simulink model "wlan_phy_rx_pmd"  (Simulink 7.8)
settings: Solver=VariableStepDiscrete  SolverMode=SingleTasking  StartTime=0.0  StopTime=rx_sim.sim_time  RelTol=1e-3
PostLoadFcn:
  | wlan_phy_rx_init
InitFcn:
  | wlan_phy_rx_init
StartFcn:
  | wlan_phy_rx_init
values from neighbouring files (symbols this model uses):
  barker_seq20 = 0.95 * circshift(barker_seq20, [0 4]) ./ max(abs(barker_seq20))    [wlan_phy_rx_init.m]
  bit_scrambler_lfsr_bytes = sum(bit_scrambler_lfsr_bytes .* repmat(2.^[0:7], 127, 1),2)    [wlan_phy_rx_init.m]
  longCorr_coef_i = [3*real(longCorr_coef)]    [wlan_phy_rx_init.m]
  longCorr_coef_q = [3*imag(longCorr_coef)]    [wlan_phy_rx_init.m]
  MAX_CP_LEN = 32    [wlan_phy_rx_init.m]
  MAX_NCBPS = NCBPS_11n_64QAM    (= 312)    [wlan_phy_rx_init.m]
  MAX_NUM_BYTES = 2^16-1    (= 65535)    [wlan_phy_rx_init.m]
  MAX_NUM_SC = 64    [wlan_phy_rx_init.m]
  MAX_NUM_SYMS = 600    [wlan_phy_rx_init.m]
  NDBPS_11a_BPSK12 = 24    [wlan_phy_rx_init.m]
  preFFT_sampBuff_numSamps = 4*MAX_NUM_SC    (= 256)    [wlan_phy_rx_init.m]
  rx_sim = struct()    [wlan_phy_rx_init.m]
  scr_ind_rev = zeros(1,128)    [wlan_phy_rx_init.m]
undefined symbols (not defined in the file or in its folder): gcbh, mcs_rom_11ag, mcs_rom_11n, wlan_rx_deinterleave_rom_gen, xlBlockLoadCallback, xlGetNormalizedPeriod, xlipgetpartsetting
libraries: xbsIndex_r4 x4943, xbsEDKLib_r4 x1
(part 4 of 28)

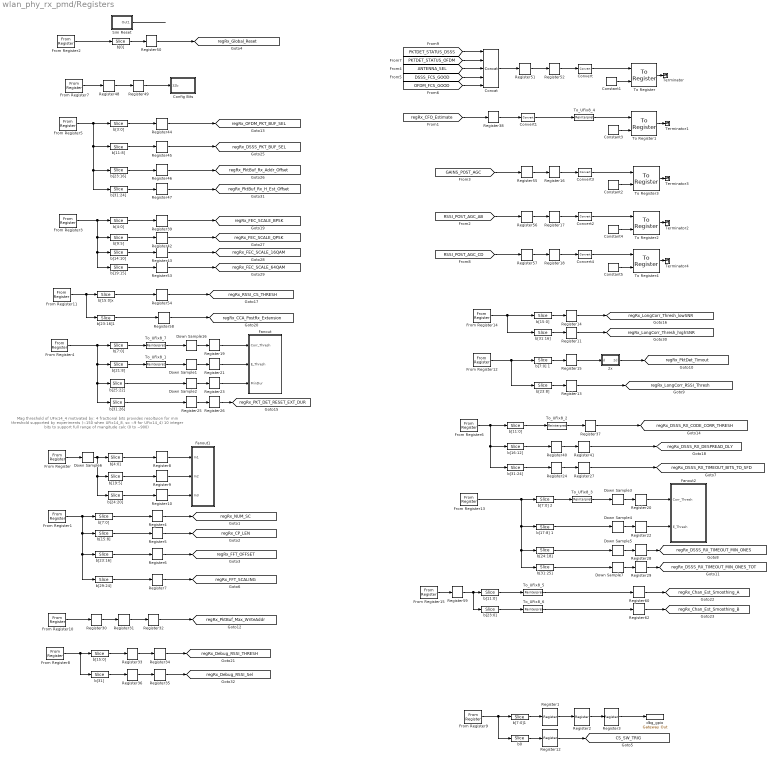
== system wlan_phy_rx_pmd/Registers ==
  note: Mag threshold of UFix14_4 motivated by:
      | 4 fractional bits provides resoltuion for min threshold supported by experiments (~150 when UFix14_8, so ~9 for UFix14_4)
      | 10 integer bits to support full range of mangitude calc (0 to ~900)
  " b[23:16] " : xbsIndex_r4/Slice  nbits=8  boolean_output=off  mode=Lower Bit Location + Width  bit1=0  base1=MSB of Input  bit0=16  base0=LSB of Input  dbl_ovrd=off  block_type=slice
      settings: block_version=VER_STRING_GOES_HERE
  " b[31:24]" : xbsIndex_r4/Slice  nbits=8  boolean_output=off  mode=Lower Bit Location + Width  bit1=0  base1=MSB of Input  bit0=24  base0=LSB of Input  dbl_ovrd=off  block_type=slice
      settings: block_version=VER_STRING_GOES_HERE
  "2x " : Subsystem
  Concat : xbsIndex_r4/Concat  num_inputs=5  dbl_ovrd=off  block_type=concat
      settings: block_version=8.2.02
  Config Bits : Subsystem
  Constant{1..5} (5 alike) : xbsIndex_r4/Constant  const=1  gui_display_data_type=Boolean  arith_type=Boolean  n_bits=30  bin_pt=0  preci_type=Single  exp_width=8  frac_width=24  explicit_period=on  period=1  equ=P=C  opselect=C  inp2=PCIN>>17  opr=+  inp1=P  carry=CIN  dbl_ovrd=off  block_type=constant
  Convert{,2..4} (4 alike) : xbsIndex_r4/Convert  gui_display_data_type=Fixed-point  arith_type=Unsigned  n_bits=32  bin_pt=0  float_type=Single  exp_bits=8  fraction_bits=24  quantization=Truncate  overflow=Wrap  en=off  latency=0  dbl_ovrd=off  pipeline=off  block_type=convert
  Convert1 : xbsIndex_r4/Convert  gui_display_data_type=Fixed-point  arith_type="Signed  (2's comp)"  n_bits=32  bin_pt=31  float_type=Single  exp_bits=8  fraction_bits=24  quantization=Truncate  overflow=Wrap  en=off  latency=0  dbl_ovrd=off  pipeline=off  block_type=convert
  Down Sample{1..5,7,16} (7 alike) : xbsIndex_r4/Down Sample  sample_ratio=8  sample_phase="Last Value of Frame  (most efficient)"  en=off  latency=1  dbl_ovrd=off  block_type=dsamp
      settings: block_version=VER_STRING_GOES_HERE
  Down Sample6 : xbsIndex_r4/Down Sample  sample_ratio=16  sample_phase="Last Value of Frame  (most efficient)"  en=off  latency=1  dbl_ovrd=off  block_type=dsamp
      settings: block_version=VER_STRING_GOES_HERE
  Fanout : Subsystem
  Fanout1 : Subsystem
  Fanout2 : Subsystem
  From Register : xbsIndex_r4/From Register  shared_memory_name='PKTDET_RSSI_CONFIG'  init=REG_RX_PKTDET_RSSI_CONFIG  period=1  ownership=Locally owned and initialized  gui_display_data_type=Fixed-point  arith_type=Unsigned  n_bits=32  bin_pt=0  preci_type=Single  dbl_ovrd=off  block_type=fromreg
      settings: AttributesFormatString=<< %<shared_memory_name> >>  infoedit="Register block that reads data to a shared memory register.  Delay of one sample period."
  From Register1 : xbsIndex_r4/From Register  shared_memory_name='FFT_Config'  init=REG_RX_FFT_Config  period=1  ownership=Locally owned and initialized  gui_display_data_type=Fixed-point  arith_type=Unsigned  n_bits=32  bin_pt=0  preci_type=Single  dbl_ovrd=off  block_type=fromreg
      settings: AttributesFormatString=<< %<shared_memory_name> >>  infoedit="Register block that reads data to a shared memory register.  Delay of one sample period."
  From Register10 : xbsIndex_r4/From Register  shared_memory_name='PKTBUF_MAX_WRITE_ADDR'  init=REG_RX_PKT_BUF_Max_Write_Addr  period=1  ownership=Locally owned and initialized  gui_display_data_type=Fixed-point  arith_type=Unsigned  n_bits=32  bin_pt=0  preci_type=Single  dbl_ovrd=off  block_type=fromreg
      settings: AttributesFormatString=<< %<shared_memory_name> >>  infoedit="Register block that reads data to a shared memory register.  Delay of one sample period."
  From Register11 : xbsIndex_r4/From Register  shared_memory_name='PHY_CCA_CONFIG'  init=REG_RX_CCA_CONFIG  period=1  ownership=Locally owned and initialized  gui_display_data_type=Fixed-point  arith_type=Unsigned  n_bits=32  bin_pt=0  preci_type=Single  dbl_ovrd=off  block_type=fromreg
      settings: AttributesFormatString=<< %<shared_memory_name> >>  infoedit="Register block that reads data to a shared memory register.  Delay of one sample period."
  From Register12 : xbsIndex_r4/From Register  shared_memory_name='LTS_Corr_Config'  init=REG_RX_LTS_Corr_Confg  period=1  ownership=Locally owned and initialized  gui_display_data_type=Fixed-point  arith_type=Unsigned  n_bits=32  bin_pt=0  preci_type=Single  dbl_ovrd=off  block_type=fromreg
      settings: AttributesFormatString=<< %<shared_memory_name> >>  infoedit="Register block that reads data to a shared memory register.  Delay of one sample period."
  From Register13 : xbsIndex_r4/From Register  shared_memory_name='PKTDET_DSSS_CONFIG'  init=REG_RX_PktDet_DSSS_Config  period=1  ownership=Locally owned and initialized  gui_display_data_type=Fixed-point  arith_type=Unsigned  n_bits=32  bin_pt=0  preci_type=Single  dbl_ovrd=off  block_type=fromreg
      settings: AttributesFormatString=<< %<shared_memory_name> >>  infoedit="Register block that reads data to a shared memory register.  Delay of one sample period."
  From Register14 : xbsIndex_r4/From Register  shared_memory_name='LTS_Corr_Thresh'  init=REG_RX_LTS_Corr_Thresh  period=1  ownership=Locally owned and initialized  gui_display_data_type=Fixed-point  arith_type=Unsigned  n_bits=32  bin_pt=0  preci_type=Single  dbl_ovrd=off  block_type=fromreg
      settings: AttributesFormatString=<< %<shared_memory_name> >>  infoedit="Register block that reads data to a shared memory register.  Delay of one sample period."
  From Register15 : xbsIndex_r4/From Register  shared_memory_name='CHAN_EST_SMOOTHING'  init=REG_RX_Chan_Est_Smoothing  period=1  ownership=Locally owned and initialized  gui_display_data_type=Fixed-point  arith_type=Unsigned  n_bits=32  bin_pt=0  preci_type=Single  dbl_ovrd=off  block_type=fromreg
      settings: AttributesFormatString=<< %<shared_memory_name> >>  infoedit="Register block that reads data to a shared memory register.  Delay of one sample period."
  From Register2 : xbsIndex_r4/From Register  shared_memory_name='Control'  init=REG_RX_Control  period=1  ownership=Locally owned and initialized  gui_display_data_type=Fixed-point  arith_type=Unsigned  n_bits=32  bin_pt=0  preci_type=Single  dbl_ovrd=off  block_type=fromreg
      settings: AttributesFormatString=<< %<shared_memory_name> >>  infoedit="Register block that reads data to a shared memory register.  Delay of one sample period."
  From Register3 : xbsIndex_r4/From Register  shared_memory_name='FEC_CONFIG'  init=REG_RX_FEC_Config  period=1  ownership=Locally owned and initialized  gui_display_data_type=Fixed-point  arith_type=Unsigned  n_bits=32  bin_pt=0  preci_type=Single  dbl_ovrd=off  block_type=fromreg
      settings: AttributesFormatString=<< %<shared_memory_name> >>  infoedit="Register block that reads data to a shared memory register.  Delay of one sample period."
  From Register4 : xbsIndex_r4/From Register  shared_memory_name='PKTDET_AUTOCORR_CONFIG'  init=REG_RX_PktDet_AutoCorr_Config  period=1  ownership=Locally owned and initialized  gui_display_data_type=Fixed-point  arith_type=Unsigned  n_bits=32  bin_pt=0  preci_type=Single  dbl_ovrd=off  block_type=fromreg
      settings: AttributesFormatString=<< %<shared_memory_name> >>  infoedit="Register block that reads data to a shared memory register.  Delay of one sample period."
  From Register5 : xbsIndex_r4/From Register  shared_memory_name='PKT_BUF_SEL'  init=REG_RX_PktBuf_Sel  period=1  ownership=Locally owned and initialized  gui_display_data_type=Fixed-point  arith_type=Unsigned  n_bits=32  bin_pt=0  preci_type=Single  dbl_ovrd=off  block_type=fromreg
      settings: AttributesFormatString=<< %<shared_memory_name> >>  infoedit="Register block that reads data to a shared memory register.  Delay of one sample period."
  From Register6 : xbsIndex_r4/From Register  shared_memory_name='DSSS_RX_CONFIG'  init=REG_RX_DSSS_RX_CONFIG  period=1  ownership=Locally owned and initialized  gui_display_data_type=Fixed-point  arith_type=Unsigned  n_bits=32  bin_pt=0  preci_type=Single  dbl_ovrd=off  block_type=fromreg
      settings: AttributesFormatString=<< %<shared_memory_name> >>  infoedit="Register block that reads data to a shared memory register.  Delay of one sample period."
  From Register7 : xbsIndex_r4/From Register  shared_memory_name='CONFIG'  init=REG_RX_Config  period=1  ownership=Locally owned and initialized  gui_display_data_type=Fixed-point  arith_type=Unsigned  n_bits=32  bin_pt=0  preci_type=Single  dbl_ovrd=off  block_type=fromreg
      settings: AttributesFormatString=<< %<shared_memory_name> >>  infoedit="Register block that reads data to a shared memory register.  Delay of one sample period."
  From Register8 : xbsIndex_r4/From Register  shared_memory_name='RSSI_THRESH'  init=600  period=1  ownership=Locally owned and initialized  gui_display_data_type=Fixed-point  arith_type=Unsigned  n_bits=32  bin_pt=0  preci_type=Single  dbl_ovrd=off  block_type=fromreg
      settings: AttributesFormatString=<< %<shared_memory_name> >>  infoedit="Register block that reads data to a shared memory register.  Delay of one sample period."
  From Register9 : xbsIndex_r4/From Register  shared_memory_name='DEBUG_GPIO'  init=0  period=1  ownership=Locally owned and initialized  gui_display_data_type=Fixed-point  arith_type=Unsigned  n_bits=32  bin_pt=0  preci_type=Single  dbl_ovrd=off  block_type=fromreg
      settings: AttributesFormatString=<< %<shared_memory_name> >>  infoedit="Register block that reads data to a shared memory register.  Delay of one sample period."
  Register{1..60,62} (61 alike) : xbsIndex_r4/Register  init=0  rst=off  en=off  dbl_ovrd=off  block_type=register
  Sim Reset : Subsystem
  Terminator{,1..4} (5 alike) : Terminator
  To Register : xbsIndex_r4/To Register  shared_memory_name='Status'  init=0  ownership=Locally owned and initialized  explicit_data_type=on  gui_display_data_type=Fixed-point  arith_type=Unsigned  n_bits=32  bin_pt=0  preci_type=Single  dbl_ovrd=off  block_type=toreg
      settings: AttributesFormatString=<< %<shared_memory_name> >>  infoedit="Register block that writes data to a shared memory register.  Delay of one sample period."
  To Register1 : xbsIndex_r4/To Register  shared_memory_name='CFO_EST_TIME_DOMAIN'  init=0  ownership=Locally owned and initialized  explicit_data_type=on  gui_display_data_type=Fixed-point  arith_type=Unsigned  n_bits=32  bin_pt=0  preci_type=Single  dbl_ovrd=off  block_type=toreg
      settings: AttributesFormatString=<< %<shared_memory_name> >>  infoedit="Register block that writes data to a shared memory register.  Delay of one sample period."
  To Register2 : xbsIndex_r4/To Register  shared_memory_name='RX_PKT_RSSI_AB'  init=0  ownership=Locally owned and initialized  explicit_data_type=on  gui_display_data_type=Fixed-point  arith_type=Unsigned  n_bits=32  bin_pt=0  preci_type=Single  dbl_ovrd=off  block_type=toreg
      settings: AttributesFormatString=<< %<shared_memory_name> >>  infoedit="Register block that writes data to a shared memory register.  Delay of one sample period."
  To Register3 : xbsIndex_r4/To Register  shared_memory_name='RX_PKT_AGC_GAINS'  init=0  ownership=Locally owned and initialized  explicit_data_type=on  gui_display_data_type=Fixed-point  arith_type=Unsigned  n_bits=32  bin_pt=0  preci_type=Single  dbl_ovrd=off  block_type=toreg
      settings: AttributesFormatString=<< %<shared_memory_name> >>  infoedit="Register block that writes data to a shared memory register.  Delay of one sample period."
  To Register4 : xbsIndex_r4/To Register  shared_memory_name='RX_PKT_RSSI_CD'  init=0  ownership=Locally owned and initialized  explicit_data_type=on  gui_display_data_type=Fixed-point  arith_type=Unsigned  n_bits=32  bin_pt=0  preci_type=Single  dbl_ovrd=off  block_type=toreg
      settings: AttributesFormatString=<< %<shared_memory_name> >>  infoedit="Register block that writes data to a shared memory register.  Delay of one sample period."
  To_UFix8_1 : xbsIndex_r4/Reinterpret  force_arith_type=on  arith_type=Unsigned  force_bin_pt=on  bin_pt=4  block_type=cast
      settings: block_version=VER_STRING_GOES_HERE
  To_UFix8_2 : xbsIndex_r4/Reinterpret  force_arith_type=on  arith_type=Unsigned  force_bin_pt=on  bin_pt=4  block_type=cast
      settings: block_version=VER_STRING_GOES_HERE
  To_UFix8_3 : xbsIndex_r4/Reinterpret  force_arith_type=on  arith_type=Unsigned  force_bin_pt=on  bin_pt=6  block_type=cast
      settings: block_version=VER_STRING_GOES_HERE
  To_UFix8_4 : xbsIndex_r4/Reinterpret  force_arith_type=on  arith_type=Unsigned  force_bin_pt=on  bin_pt=0  block_type=cast
      settings: block_version=VER_STRING_GOES_HERE
  To_UFix8_5 : xbsIndex_r4/Reinterpret  force_arith_type=on  arith_type=Unsigned  force_bin_pt=on  bin_pt=12  block_type=cast
      settings: block_version=VER_STRING_GOES_HERE
  To_UFix8_6 : xbsIndex_r4/Reinterpret  force_arith_type=on  arith_type=Unsigned  force_bin_pt=on  bin_pt=12  block_type=cast
      settings: block_version=VER_STRING_GOES_HERE
  To_UFix8_7 : xbsIndex_r4/Reinterpret  force_arith_type=on  arith_type=Unsigned  force_bin_pt=on  bin_pt=8  block_type=cast
      settings: block_version=VER_STRING_GOES_HERE
  b0 : xbsIndex_r4/Slice  nbits=1  boolean_output=off  mode=Lower Bit Location + Width  bit1=0  base1=MSB of Input  bit0=0  base0=LSB of Input  dbl_ovrd=off  block_type=slice
      settings: block_version=VER_STRING_GOES_HERE
  b[0] : xbsIndex_r4/Slice  nbits=1  boolean_output=off  mode=Lower Bit Location + Width  bit1=0  base1=MSB of Input  bit0=0  base0=LSB of Input  dbl_ovrd=off  block_type=slice
      settings: block_version=VER_STRING_GOES_HERE
  b[11:0] : xbsIndex_r4/Slice  nbits=12  boolean_output=off  mode=Lower Bit Location + Width  bit1=0  base1=MSB of Input  bit0=0  base0=LSB of Input  dbl_ovrd=off  block_type=slice
      settings: block_version=VER_STRING_GOES_HERE
  "b[11:0]   " : xbsIndex_r4/Slice  nbits=12  boolean_output=off  mode=Lower Bit Location + Width  bit1=0  base1=MSB of Input  bit0=0  base0=LSB of Input  dbl_ovrd=off  block_type=slice
      settings: block_version=VER_STRING_GOES_HERE
  b[11:8] : xbsIndex_r4/Slice  nbits=4  boolean_output=off  mode=Lower Bit Location + Width  bit1=0  base1=MSB of Input  bit0=8  base0=LSB of Input  dbl_ovrd=off  block_type=slice
      settings: block_version=VER_STRING_GOES_HERE
  b[14:10] : xbsIndex_r4/Slice  nbits=5  boolean_output=off  mode=Lower Bit Location + Width  bit1=0  base1=MSB of Input  bit0=10  base0=LSB of Input  dbl_ovrd=off  block_type=slice
      settings: block_version=VER_STRING_GOES_HERE
  b[15:0] : xbsIndex_r4/Slice  nbits=16  boolean_output=off  mode=Lower Bit Location + Width  bit1=0  base1=MSB of Input  bit0=0  base0=LSB of Input  dbl_ovrd=off  block_type=slice
      settings: block_version=VER_STRING_GOES_HERE
  "b[15:0]    " : xbsIndex_r4/Slice  nbits=16  boolean_output=off  mode=Lower Bit Location + Width  bit1=0  base1=MSB of Input  bit0=0  base0=LSB of Input  dbl_ovrd=off  block_type=slice
      settings: block_version=VER_STRING_GOES_HERE
  b[15:0]x : xbsIndex_r4/Slice  nbits=16  boolean_output=off  mode=Lower Bit Location + Width  bit1=0  base1=MSB of Input  bit0=0  base0=LSB of Input  dbl_ovrd=off  block_type=slice
      settings: block_version=VER_STRING_GOES_HERE
  b[15:8] : xbsIndex_r4/Slice  nbits=8  boolean_output=off  mode=Lower Bit Location + Width  bit1=0  base1=MSB of Input  bit0=8  base0=LSB of Input  dbl_ovrd=off  block_type=slice
      settings: block_version=VER_STRING_GOES_HERE
  "b[16:12]   " : xbsIndex_r4/Slice  nbits=5  boolean_output=off  mode=Lower Bit Location + Width  bit1=0  base1=MSB of Input  bit0=12  base0=LSB of Input  dbl_ovrd=off  block_type=slice
      settings: block_version=VER_STRING_GOES_HERE
  b[17:8] 1 : xbsIndex_r4/Slice  nbits=10  boolean_output=off  mode=Lower Bit Location + Width  bit1=0  base1=MSB of Input  bit0=8  base0=LSB of Input  dbl_ovrd=off  block_type=slice
      settings: block_version=VER_STRING_GOES_HERE
  b[19:15] : xbsIndex_r4/Slice  nbits=5  boolean_output=off  mode=Lower Bit Location + Width  bit1=0  base1=MSB of Input  bit0=15  base0=LSB of Input  dbl_ovrd=off  block_type=slice
      settings: block_version=VER_STRING_GOES_HERE
  b[19:5] : xbsIndex_r4/Slice  nbits=15  boolean_output=off  mode=Lower Bit Location + Width  bit1=0  base1=MSB of Input  bit0=5  base0=LSB of Input  dbl_ovrd=off  block_type=slice
      settings: block_version=VER_STRING_GOES_HERE
  "b[21:8] " : xbsIndex_r4/Slice  nbits=14  boolean_output=off  mode=Lower Bit Location + Width  bit1=0  base1=MSB of Input  bit0=8  base0=LSB of Input  dbl_ovrd=off  block_type=slice
      settings: block_version=VER_STRING_GOES_HERE
  b[23:0] : xbsIndex_r4/Slice  nbits=12  boolean_output=off  mode=Lower Bit Location + Width  bit1=0  base1=MSB of Input  bit0=12  base0=LSB of Input  dbl_ovrd=off  block_type=slice
      settings: block_version=VER_STRING_GOES_HERE
  b[23:16] : xbsIndex_r4/Slice  nbits=8  boolean_output=off  mode=Lower Bit Location + Width  bit1=0  base1=MSB of Input  bit0=16  base0=LSB of Input  dbl_ovrd=off  block_type=slice
      settings: block_version=VER_STRING_GOES_HERE
  b[23:16]1 : xbsIndex_r4/Slice  nbits=8  boolean_output=off  mode=Lower Bit Location + Width  bit1=0  base1=MSB of Input  bit0=16  base0=LSB of Input  dbl_ovrd=off  block_type=slice
      settings: block_version=VER_STRING_GOES_HERE
  b[23:8] : xbsIndex_r4/Slice  nbits=16  boolean_output=off  mode=Lower Bit Location + Width  bit1=0  base1=MSB of Input  bit0=8  base0=LSB of Input  dbl_ovrd=off  block_type=slice
      settings: block_version=VER_STRING_GOES_HERE
  b[24:18] : xbsIndex_r4/Slice  nbits=7  boolean_output=off  mode=Lower Bit Location + Width  bit1=0  base1=MSB of Input  bit0=18  base0=LSB of Input  dbl_ovrd=off  block_type=slice
      settings: block_version=VER_STRING_GOES_HERE
  b[24:20] : xbsIndex_r4/Slice  nbits=5  boolean_output=off  mode=Lower Bit Location + Width  bit1=0  base1=MSB of Input  bit0=20  base0=LSB of Input  dbl_ovrd=off  block_type=slice
      settings: block_version=VER_STRING_GOES_HERE
  "b[25:22] " : xbsIndex_r4/Slice  nbits=4  boolean_output=off  mode=Lower Bit Location + Width  bit1=0  base1=MSB of Input  bit0=22  base0=LSB of Input  dbl_ovrd=off  block_type=slice
      settings: block_version=VER_STRING_GOES_HERE
  b[29:24] : xbsIndex_r4/Slice  nbits=6  boolean_output=off  mode=Lower Bit Location + Width  bit1=0  base1=MSB of Input  bit0=24  base0=LSB of Input  dbl_ovrd=off  block_type=slice
      settings: block_version=VER_STRING_GOES_HERE
  b[31:16] : xbsIndex_r4/Slice  nbits=16  boolean_output=off  mode=Lower Bit Location + Width  bit1=0  base1=MSB of Input  bit0=16  base0=LSB of Input  dbl_ovrd=off  block_type=slice
      settings: block_version=VER_STRING_GOES_HERE
  b[31:24] : xbsIndex_r4/Slice  nbits=8  boolean_output=off  mode=Lower Bit Location + Width  bit1=0  base1=MSB of Input  bit0=24  base0=LSB of Input  dbl_ovrd=off  block_type=slice
      settings: block_version=VER_STRING_GOES_HERE
  b[31:25] : xbsIndex_r4/Slice  nbits=7  boolean_output=off  mode=Lower Bit Location + Width  bit1=0  base1=MSB of Input  bit0=25  base0=LSB of Input  dbl_ovrd=off  block_type=slice
      settings: block_version=VER_STRING_GOES_HERE
  b[31:26] : xbsIndex_r4/Slice  nbits=6  boolean_output=off  mode=Lower Bit Location + Width  bit1=0  base1=MSB of Input  bit0=26  base0=LSB of Input  dbl_ovrd=off  block_type=slice
      settings: block_version=VER_STRING_GOES_HERE
  b[31] : xbsIndex_r4/Slice  nbits=1  boolean_output=off  mode=Lower Bit Location + Width  bit1=0  base1=MSB of Input  bit0=31  base0=LSB of Input  dbl_ovrd=off  block_type=slice
      settings: block_version=VER_STRING_GOES_HERE
  b[3:0] : xbsIndex_r4/Slice  nbits=4  boolean_output=off  mode=Lower Bit Location + Width  bit1=0  base1=MSB of Input  bit0=0  base0=LSB of Input  dbl_ovrd=off  block_type=slice
      settings: block_version=VER_STRING_GOES_HERE
  b[4:0] : xbsIndex_r4/Slice  nbits=5  boolean_output=off  mode=Lower Bit Location + Width  bit1=0  base1=MSB of Input  bit0=0  base0=LSB of Input  dbl_ovrd=off  block_type=slice
      settings: block_version=VER_STRING_GOES_HERE
  "b[4:0]  " : xbsIndex_r4/Slice  nbits=5  boolean_output=off  mode=Lower Bit Location + Width  bit1=0  base1=MSB of Input  bit0=0  base0=LSB of Input  dbl_ovrd=off  block_type=slice
      settings: block_version=VER_STRING_GOES_HERE
  b[7:0] : xbsIndex_r4/Slice  nbits=8  boolean_output=off  mode=Lower Bit Location + Width  bit1=0  base1=MSB of Input  bit0=0  base0=LSB of Input  dbl_ovrd=off  block_type=slice
      settings: block_version=VER_STRING_GOES_HERE
  "b[7:0] " : xbsIndex_r4/Slice  nbits=8  boolean_output=off  mode=Lower Bit Location + Width  bit1=0  base1=MSB of Input  bit0=0  base0=LSB of Input  dbl_ovrd=off  block_type=slice
      settings: block_version=VER_STRING_GOES_HERE
  b[7:0] 1 : xbsIndex_r4/Slice  nbits=8  boolean_output=off  mode=Lower Bit Location + Width  bit1=0  base1=MSB of Input  bit0=0  base0=LSB of Input  dbl_ovrd=off  block_type=slice
      settings: block_version=VER_STRING_GOES_HERE
  b[7:0] 2 : xbsIndex_r4/Slice  nbits=8  boolean_output=off  mode=Lower Bit Location + Width  bit1=0  base1=MSB of Input  bit0=0  base0=LSB of Input  dbl_ovrd=off  block_type=slice
      settings: block_version=VER_STRING_GOES_HERE
  b[7:0]1 : xbsIndex_r4/Slice  nbits=8  boolean_output=off  mode=Lower Bit Location + Width  bit1=0  base1=MSB of Input  bit0=0  base0=LSB of Input  dbl_ovrd=off  block_type=slice
      settings: block_version=VER_STRING_GOES_HERE
  b[9:5] : xbsIndex_r4/Slice  nbits=5  boolean_output=off  mode=Lower Bit Location + Width  bit1=0  base1=MSB of Input  bit0=5  base0=LSB of Input  dbl_ovrd=off  block_type=slice
      settings: block_version=VER_STRING_GOES_HERE
  dbg_gpio : xbsIndex_r4/Gateway Out  inherit_from_input=off  hdl_port=on  timing_constraint=None  locs_specified=off  LOCs={}  UseAsDAC=off  DACChannel='1'  block_type=gatewayout
  tag scope: CS_SW_TRIG=global, regRx_CCA_PostRx_Extension=global, regRx_CP_LEN=global, regRx_Chan_Est_Smoothing_A=global, regRx_Chan_Est_Smoothing_B=global, regRx_DSSS_PKT_BUF_SEL=global, regRx_DSSS_RX_CODE_CORR_THRESH=global, regRx_DSSS_RX_DESPREAD_DLY=global, regRx_DSSS_RX_TIMEOUT_BITS_TO_SFD=global, regRx_DSSS_RX_TIMEOUT_MIN_ONES=global, regRx_DSSS_RX_TIMEOUT_MIN_ONES_TOT=global, regRx_Debug_RSSI_Sel=global, regRx_Debug_RSSI_THRESH=global, regRx_FEC_SCALE_16QAM=global, regRx_FEC_SCALE_64QAM=global, regRx_FEC_SCALE_BPSK=global, regRx_FEC_SCALE_QPSK=global, regRx_FFT_OFFSET=global, regRx_FFT_SCALING=global, regRx_Global_Reset=global, regRx_LongCorr_RSSI_Thresh=global, regRx_LongCorr_Thresh_highSNR=global, regRx_LongCorr_Thresh_lowSNR=global, regRx_NUM_SC=global, regRx_OFDM_PKT_BUF_SEL=global, regRx_PKT_DET_RESET_EXT_DUR=global, regRx_PktBuf_Max_WriteAddr=global, regRx_PktBuf_Rx_Addr_Offset=global, regRx_PktBuf_Rx_H_Est_Offset=global, regRx_PktDet_Timout=global, regRx_RSSI_CS_THRESH=global
  wires:
  b[31:16]/1 -> Register11/1
  From Register1/1 -> b[7:0]/1, b[15:8]/1, b[23:16]/1, b[29:24]/1
  b[7:0]/1 -> Register4/1
  b[15:8]/1 -> Register5/1
  b[23:16]/1 -> Register6/1
  From Register2/1 -> b[0]/1
  b[0]/1 -> Register50/1
  From Register3/1 -> "b[4:0]  "/1, b[9:5]/1, b[14:10]/1, b[19:15]/1
  b[29:24]/1 -> Register7/1
  [DSSS_FCS_GOOD] -> Concat/4
  [OFDM_FCS_GOOD] -> Concat/5
  Constant1/1 -> To Register/2
  To Register/1 -> Terminator/1
  From Register4/1 -> "b[7:0] "/1, "b[21:8] "/1, "b[25:22] "/1, b[31:26]/1
  "b[7:0] "/1 -> To_UFix8_7/1
  "b[21:8] "/1 -> To_UFix8_1/1
  To_UFix8_7/1 -> Down Sample16/1
  To_UFix8_1/1 -> Down Sample1/1
  Down Sample16/1 -> Register19/1
  Down Sample1/1 -> Register21/1
  b[15:0]/1 -> Register14/1
  "b[25:22] "/1 -> Down Sample2/1
  Down Sample2/1 -> Register23/1
  Sim Reset/1(Out1) -> ?
  From Register5/1 -> b[3:0]/1, b[11:8]/1, " b[23:16] "/1, " b[31:24]"/1
  b[3:0]/1 -> Register44/1
  From Register6/1 -> "b[11:0]   "/1, "b[16:12]   "/1, b[31:24]/1
  "b[11:0]   "/1 -> To_UFix8_2/1
  To_UFix8_2/1 -> Register37/1
  From Register8/1 -> "b[15:0]    "/1, b[31]/1
  "b[15:0]    "/1 -> Register33/1
  From Register9/1 -> b[7:0]1/1, b0/1
  b[7:0]1/1 -> Register1/1
  Register1/1 -> Register2/1
  Register2/1 -> Register3/1
  Register3/1 -> dbg_gpio/1
  b[4:0]/1 -> Register8/1
  Down Sample6/1 -> b[4:0]/1, b[19:5]/1, b[24:20]/1
  b[19:5]/1 -> Register9/1
  From Register/1 -> Down Sample6/1
  b[24:20]/1 -> Register10/1
  From Register7/1 -> Register48/1
  Register4/1 -> [regRx_NUM_SC]
  Register5/1 -> [regRx_CP_LEN]
  Register6/1 -> [regRx_FFT_OFFSET]
  Register7/1 -> [regRx_FFT_SCALING]
  Register8/1 -> Fanout1/1(In1)
  Register9/1 -> Fanout1/2(In2)
  Register10/1 -> Fanout1/3(In3)
  Register11/1 -> [regRx_LongCorr_Thresh_highSNR]
  b[31:26]/1 -> Register25/1
  From Register11/1 -> b[15:0]x/1, b[23:16]1/1
  b[15:0]x/1 -> Register54/1
  b[23:16]1/1 -> Register58/1
  b[11:8]/1 -> Register45/1
  Convert/1 -> To Register/1
  " b[23:16] "/1 -> Register46/1
  "b[4:0]  "/1 -> Register39/1
  b[9:5]/1 -> Register42/1
  b[14:10]/1 -> Register43/1
  b0/1 -> Register12/1
  Register12/1 -> [CS_SW_TRIG]
  Constant4/1 -> To Register2/2
  To Register2/1 -> Terminator2/1
  Convert2/1 -> To Register2/1
  Register13/1 -> [regRx_LongCorr_RSSI_Thresh]
  b[23:8]/1 -> Register13/1
  Register14/1 -> [regRx_LongCorr_Thresh_lowSNR]
  From Register14/1 -> b[15:0]/1, b[31:16]/1
  From Register12/1 -> b[7:0] 1/1, b[23:8]/1
  "2x "/1(2d) -> [regRx_PktDet_Timout]
  Register15/1 -> "2x "/1(d)
  b[7:0] 1/1 -> Register15/1
  " b[31:24]"/1 -> Register47/1
  [RSSI_POST_AGC_AB] -> Register56/1
  [ANTENNA_SEL] -> Concat/3
  Constant2/1 -> To Register3/2
  To Register3/1 -> Terminator3/1
  Convert3/1 -> To Register3/1
  [GAINS_POST_AGC] -> Register55/1
  Register16/1 -> Convert3/1
  Register17/1 -> Convert2/1
  b[31]/1 -> Register36/1
  Concat/1 -> Register51/1
  [PKTDET_STATUS_OFDM] -> Concat/2
  Constant5/1 -> To Register4/2
  To Register4/1 -> Terminator4/1
  Convert4/1 -> To Register4/1
  Register18/1 -> Convert4/1
  [RSSI_POST_AGC_CD] -> Register57/1
  Register19/1 -> Fanout/1(Corr_Thresh)
  Register21/1 -> Fanout/2(E_Thresh)
  Register23/1 -> Fanout/3(MinDur)
  Register33/1 -> Register34/1
  Register34/1 -> [regRx_Debug_RSSI_THRESH]
  Register35/1 -> [regRx_Debug_RSSI_Sel]
  Register36/1 -> Register35/1
  Register37/1 -> [regRx_DSSS_RX_CODE_CORR_THRESH]
  Register40/1 -> Register41/1
  Register41/1 -> [regRx_DSSS_RX_DESPREAD_DLY]
  Register44/1 -> [regRx_OFDM_PKT_BUF_SEL]
  Register45/1 -> [regRx_DSSS_PKT_BUF_SEL]
  Register46/1 -> [regRx_PktBuf_Rx_Addr_Offset]
  Register47/1 -> [regRx_PktBuf_Rx_H_Est_Offset]
  Register48/1 -> Register49/1
  Register49/1 -> Config Bits/1(32b)
  Register50/1 -> [regRx_Global_Reset]
  Register51/1 -> Register52/1
  Register52/1 -> Convert/1
  Register55/1 -> Register16/1
  Register56/1 -> Register17/1
  Register57/1 -> Register18/1
  Register25/1 -> Register26/1
  Register26/1 -> [regRx_PKT_DET_RESET_EXT_DUR]
  From Register13/1 -> b[7:0] 2/1, b[17:8] 1/1, b[24:18]/1, b[31:25]/1
  b[7:0] 2/1 -> To_UFix8_3/1
  To_UFix8_3/1 -> Down Sample3/1
  Down Sample3/1 -> Register20/1
  Register20/1 -> Fanout2/1(Corr_Thresh)
  b[17:8] 1/1 -> Down Sample4/1
  Down Sample4/1 -> Register22/1
  Register22/1 -> Fanout2/2(E_Thresh)
  [PKTDET_STATUS_DSSS] -> Concat/1
  "b[16:12]   "/1 -> Register40/1
  b[31:24]/1 -> Register24/1
  Register24/1 -> Register27/1
  Register27/1 -> [regRx_DSSS_RX_TIMEOUT_BITS_TO_SFD]
  b[24:18]/1 -> Down Sample5/1
  Down Sample5/1 -> Register28/1
  Register28/1 -> [regRx_DSSS_RX_TIMEOUT_MIN_ONES]
  b[31:25]/1 -> Down Sample7/1
  Down Sample7/1 -> Register29/1
  Register29/1 -> [regRx_DSSS_RX_TIMEOUT_MIN_ONES_TOT]
  From Register10/1 -> Register30/1
  Register30/1 -> Register31/1
  Register31/1 -> Register32/1
  Register32/1 -> [regRx_PktBuf_Max_WriteAddr]
  [regRx_CFO_Estimate] -> Register38/1
  Constant3/1 -> To Register1/2
  To Register1/1 -> Terminator1/1
  To_UFix8_4/1 -> To Register1/1
  Convert1/1 -> To_UFix8_4/1
  Register38/1 -> Convert1/1
  b[19:15]/1 -> Register53/1
  Register39/1 -> [regRx_FEC_SCALE_BPSK]
  Register42/1 -> [regRx_FEC_SCALE_QPSK]
  Register43/1 -> [regRx_FEC_SCALE_16QAM]
  Register53/1 -> [regRx_FEC_SCALE_64QAM]
  Register54/1 -> [regRx_RSSI_CS_THRESH]
  Register58/1 -> [regRx_CCA_PostRx_Extension]
  From Register15/1 -> Register59/1
  Register59/1 -> b[11:0]/1, b[23:0]/1
  b[11:0]/1 -> To_UFix8_5/1
  b[23:0]/1 -> To_UFix8_6/1
  To_UFix8_5/1 -> Register60/1
  To_UFix8_6/1 -> Register62/1
  Register60/1 -> [regRx_Chan_Est_Smoothing_A]
  Register62/1 -> [regRx_Chan_Est_Smoothing_B]

== system wlan_phy_rx_pmd/Resets ==
  Convert1 : xbsIndex_r4/Convert  gui_display_data_type=Boolean  arith_type=Boolean  n_bits=16  bin_pt=14  float_type=Single  exp_bits=8  fraction_bits=24  quantization=Truncate  overflow=Wrap  en=off  latency=0  dbl_ovrd=off  pipeline=off  block_type=convert
  tag scope: RX_GLOBAL_RESET=global
  wires:
  Convert1/1 -> [RX_GLOBAL_RESET]
  [regRx_Global_Reset] -> Convert1/1

== system wlan_phy_rx_pmd/Sync & Antenna Sel ==
  Rx I : Inport
  Rx Q : Inport  Port=2
  IQ Valid : Inport  Port=3
  RSSI : Inport  Port=4
  "Reset " : Inport  Port=5
  ADC/Corr : Scope  NumInputPorts=10
      settings: TimeRange=13944  YMin=0.95~-1~-1~0.95~-1~-1~-1~-1~-1~-1  YMax=1.05~1~1~1.05~1~1~1~1~1~1  SaveName=ScopeData34
  Antenna Selection : Subsystem
  Debug  Outputs : Subsystem
  Gateway Out{,1..4} (5 alike) : xbsIndex_r4/Gateway Out  inherit_from_input=off  hdl_port=off  timing_constraint=None  locs_specified=off  LOCs={}  UseAsDAC=off  DACChannel='1'  block_type=gatewayout
  LTS Correlation : Subsystem
  PHY CCA : Subsystem
  Pkt Det : Subsystem
  Pkt Det AGC Ctrl : Subsystem
  Resets : Subsystem
  " Pkt Det DSSS" : Outport
  " Rx I" : Outport  Port=2
  " Rx Q" : Outport  Port=3
  " IQ Valid" : Outport  Port=4
  " Pkt Det OFDM" : Outport  Port=5
  LTS Sync : Outport  Port=6
  wires:
  LTS Correlation/1(Det) -> LTS Sync/1, PHY CCA/1(LTS Sync), Debug  Outputs/1(LTS)
  LTS Correlation/2(Timeout) -> PHY CCA/2(LTS Timeout), Resets/3(LTS Timeout), Debug  Outputs/2(LTS Timeout), Gateway Out2/1
  Gateway Out2/1 -> ADC/Corr/3  "LTS TO"
  Gateway Out1/1 -> ADC/Corr/2  "Pkt Done"
  Gateway Out/1 -> ADC/Corr/1  "Pkt Det OFDM"
  Pkt Det/1(Det OFDM) -> Antenna Selection/1(Pkt Det), LTS Correlation/5(En), PHY CCA/4(Det OFDM), Debug  Outputs/3(Det OFDM), Gateway Out/1, " Pkt Det OFDM"/1, Pkt Det AGC Ctrl/1(Det OFDM)
  Resets/1(" OFDM Reset") -> Antenna Selection/6(Reset), LTS Correlation/6(Reset), PHY CCA/6(Reset OFDM), Gateway Out1/1, Pkt Det/1(Reset OFDM)
  IQ Valid/1 -> Pkt Det/5(IQ Valid), Antenna Selection/4(IQ Valid)
  RSSI/1 -> Pkt Det/6(RSSI Vec), Antenna Selection/5(RSSI Vec), PHY CCA/5(Avg RSSI Vec)
  Rx I/1 -> Pkt Det/3(Rx I Vec), Antenna Selection/2(Rx I Vec)
  Rx Q/1 -> Pkt Det/4(Rx Q Vec), Antenna Selection/3(Rx Q Vec)
  Antenna Selection/1(Rx I) -> " Rx I"/1, LTS Correlation/1(rx_I)
  Antenna Selection/2(" Rx Q") -> " Rx Q"/1, LTS Correlation/2(rx_Q)
  Antenna Selection/3(" IQ Valid") -> " IQ Valid"/1, LTS Correlation/3(rx_IQ_valid), PHY CCA/3(IQ Valid)
  Antenna Selection/4(RSSI) -> LTS Correlation/4(RSSI), Gateway Out3/1  "Avg RSSI"
  Gateway Out3/1 -> ADC/Corr/9
  Pkt Det/2(Det DSSS) -> Gateway Out4/1, " Pkt Det DSSS"/1, Pkt Det AGC Ctrl/2(Det DSSS), Debug  Outputs/4(Det DSSS), PHY CCA/7(Det DSSS)
  Gateway Out4/1 -> ADC/Corr/4  "Pkt Det DSSS"
  Resets/2(DSSS Reset) -> Pkt Det/2(Reset DSSS), PHY CCA/8(Reset DSSS)
  [DSSS_RX_RESET] -> Resets/2("DSSS Reset ")
  "Reset "/1 -> Resets/1(OFDM Reset)

== system wlan_phy_rx_pmd/CFO & Samp Buffer/CFO Correction ==
  note: DDS requires >1 cycle EN early to get setup
      | Use gloabl reset for this
      | Safe, since DDS output is ignored when
      | it's not being used, so bogus outputs
      | between packets is no problem
      |
      | DDS updates once per input sample
      | Since the FFT only consumes 64 samples
      | per OFDM symbol (ignoring the CP),
      | the DDS keeps running after each FFT
      | frame is loaded. This is fine, since the FFT is
      | configured for non-streaming (it ignores its
      | I/Q inputs while computing a transform)
  "I " : Inport
  "Q " : Inport  Port=2
  DDS Run : Inport  Port=3
  Valid : Inport  Port=4
  tlast : Inport  Port=5
  CFO Est : Inport  Port=6
  CFO Corr : Scope  NumInputPorts=8
      settings: TimeRange=35000  YMin=0~-0.4~0~-1~0~0~-0.4~0  YMax=1~0.4~1~2~1~1~0.4~1  SaveName=ScopeData3
  "Complex Multiplier 5.0 " : xbsIndex_r4/Complex Multiplier 5.0   hasatlast=off  hasatuser=on  atuserwidth=1  hasbtlast=off  hasbtuser=off  btuserwidth=1  multtype=Use_Mults  optimizegoal=Performance  flowcontrol=NonBlocking  outputwidth=16  roundmode=Truncate  hasctrltlast=off  hasctrltuser=off  ctrltuserwidth=1  outtlastbehv=Null  latencyconfig=Manual  minimumlatency=2  aclken=off  aresetn=off  trim_axipin_name=on  aportwidth=63  bportwidth=63  ip_name=Complex Multiplier  ip_version=5.0  dsptool_ready=true  ipcore_usecache=true  ipcore_useipmodelcache=true  ipcore_verbose=false  ipcore_latency_parameter='minimumlatency'  wrapper_available=true  port_translation_map={ 'aclken' => 'en', 'aresetn' => 'rst'}  structural_sim=false  block_type=cmpy_v5_0
  Constant1 : xbsIndex_r4/Constant  const=1  gui_display_data_type=Boolean  arith_type=Boolean  n_bits=16  bin_pt=14  preci_type=Single  exp_width=8  frac_width=24  explicit_period=off  period=8  equ=P=C  opselect=C  inp2=PCIN>>17  opr=+  inp1=P  carry=CIN  dbl_ovrd=off  block_type=constant
  Constant2 : xbsIndex_r4/Constant  const=1  gui_display_data_type=Fixed-point  arith_type=Signed (2's comp)  n_bits=16  bin_pt=15  preci_type=Single  exp_width=8  frac_width=24  explicit_period=on  period=1  equ=P=C  opselect=C  inp2=PCIN>>17  opr=+  inp1=P  carry=CIN  dbl_ovrd=off  block_type=constant
  Constant3 : xbsIndex_r4/Constant  const=0  gui_display_data_type=Fixed-point  arith_type=Signed (2's comp)  n_bits=16  bin_pt=15  preci_type=Single  exp_width=8  frac_width=24  explicit_period=on  period=1  equ=P=C  opselect=C  inp2=PCIN>>17  opr=+  inp1=P  carry=CIN  dbl_ovrd=off  block_type=constant
  Constant5 : xbsIndex_r4/Constant  const=1  gui_display_data_type=Boolean  arith_type=Boolean  n_bits=16  bin_pt=14  preci_type=Single  exp_width=8  frac_width=24  explicit_period=off  period=8  equ=P=C  opselect=C  inp2=PCIN>>17  opr=+  inp1=P  carry=CIN  dbl_ovrd=off  block_type=constant
  Convert1 : xbsIndex_r4/Convert  gui_display_data_type=Fixed-point  arith_type="Signed  (2's comp)"  n_bits=16  bin_pt=15  float_type=Single  exp_bits=8  fraction_bits=24  quantization=Truncate  overflow=Wrap  en=off  latency=0  dbl_ovrd=off  pipeline=off  block_type=convert
  Convert2 : xbsIndex_r4/Convert  gui_display_data_type=Fixed-point  arith_type="Signed  (2's comp)"  n_bits=16  bin_pt=15  float_type=Single  exp_bits=8  fraction_bits=24  quantization=Truncate  overflow=Wrap  en=off  latency=0  dbl_ovrd=off  pipeline=off  block_type=convert
  Convert3 : xbsIndex_r4/Convert  gui_display_data_type=Fixed-point  arith_type="Signed  (2's comp)"  n_bits=26  bin_pt=26  float_type=Single  exp_bits=8  fraction_bits=24  quantization=Truncate  overflow=Flag as error  en=off  latency=0  dbl_ovrd=off  pipeline=off  block_type=convert
  Convert4 : xbsIndex_r4/Convert  gui_display_data_type=Fixed-point  arith_type=Unsigned  n_bits=1  bin_pt=0  float_type=Single  exp_bits=8  fraction_bits=24  quantization=Truncate  overflow=Flag as error  en=off  latency=0  dbl_ovrd=off  pipeline=off  block_type=convert
  Convert5 : xbsIndex_r4/Convert  gui_display_data_type=Boolean  arith_type=Boolean  n_bits=1  bin_pt=0  float_type=Single  exp_bits=8  fraction_bits=24  quantization=Truncate  overflow=Flag as error  en=off  latency=0  dbl_ovrd=off  pipeline=off  block_type=convert
  DDS Compiler 4.0 : xbsIndex_r4/DDS Compiler 4.0   partspresent=Phase_Generator_and_SIN_COS_LUT  dds_clock_rate=160  channels=1  parameter_entry=Hardware_Parameters  spurious_free_dynamic_range=36  frequency_resolution=0.4  noise_shaping=None  phase_width=26  output_width=16  output_selection=Sine_and_Cosine  negative_sine=off  negative_cosine=off  amplitude_mode=Full_Range  memory_type=Auto  optimization_goal=Auto  dsp48_use=Minimal  latency_configuration=Auto  latency=6  has_phase_out=off  sclr_pin=off  clock_enable=on  rfd=off  rdy=on  channel_pin=off  explicit_period=off  period=1  phase_increment=Programmable  output_frequency1=0  output_frequency2=0  output_frequency3=0  output_frequency4=0  output_frequency5=0  output_frequency6=0  output_frequency7=0  output_frequency8=0  output_frequency9=0  output_frequency10=0  output_frequency11=0  output_frequency12=0  output_frequency13=0  output_frequency14=0  output_frequency15=0  output_frequency16=0  pinc1='0'  pinc2='0'  pinc3='0'  pinc4='0'  pinc5='0'  pinc6='0'  pinc7='0'  pinc8='0'  pinc9='0'  pinc10='0'  pinc11='0'  pinc12='0'  pinc13='0'  pinc14='0'  pinc15='0'  pinc16='0'  phase_offset=None  phase_offset_angles1=0  phase_offset_angles2=0  phase_offset_angles3=0  phase_offset_angles4=0  phase_offset_angles5=0  phase_offset_angles6=0  phase_offset_angles7=0  phase_offset_angles8=0  phase_offset_angles9=0  phase_offset_angles10=0  phase_offset_angles11=0  phase_offset_angles12=0  phase_offset_angles13=0  phase_offset_angles14=0  phase_offset_angles15=0  phase_offset_angles16=0  poff1='0'  poff2='0'  poff3='0'  poff4='0'  poff5='0'  poff6='0'  poff7='0'  poff8='0'  poff9='0'  poff10='0'  poff11='0'  poff12='0'  poff13='0'  poff14='0'  poff15='0'  poff16='0'  por_mode=false  gui_behaviour=Sysgen  ip_name=DDS Compiler  ip_version=4.0  dsptool_ready=true  wrapper_available=true  port_translation_map={ 'ce' => 'en', 'sclr' => 'rst' }  ipcore_xco_need_fpga_part=true  ipcore_fpga_part=xlipgetpartsetting(gcb, {'virtex4', 'xc4vsx35', '-10', 'ff668'})  ipcore_usecache=true  ipcore_useipmodelcache=true  ipcore_verbose=false  block_type=dds_compiler_v4_0
  Gateway Out{1..7,17} (8 alike) : xbsIndex_r4/Gateway Out  inherit_from_input=off  hdl_port=off  timing_constraint=None  locs_specified=off  LOCs={}  UseAsDAC=off  DACChannel='1'  block_type=gatewayout
  Logical2 : xbsIndex_r4/Logical  logical_function=OR  inputs=3  en=off  latency=0  precision=Full  arith_type=Unsigned  n_bits=16  bin_pt=0  align_bp=on  dbl_ovrd=off  block_type=logical
  Sim Reset : Subsystem
  valid : Outport
  I : Outport  Port=2
  Q : Outport  Port=3
  " tlast" : Outport  Port=4
  wires:
  Constant1/1 -> DDS Compiler 4.0/1
  CFO Est/1 -> Convert3/1
  DDS Run/1 -> Logical2/1
  "Complex Multiplier 5.0 "/2 -> Convert2/1, Gateway Out6/1
  "Complex Multiplier 5.0 "/3 -> Convert1/1
  "I "/1 -> "Complex Multiplier 5.0 "/3, Gateway Out1/1
  "Q "/1 -> "Complex Multiplier 5.0 "/2
  Gateway Out17/1 -> CFO Corr/1
  Gateway Out1/1 -> CFO Corr/2
  Gateway Out2/1 -> CFO Corr/3
  Gateway Out3/1 -> CFO Corr/4
  Gateway Out4/1 -> CFO Corr/5
  DDS Compiler 4.0/2 -> "Complex Multiplier 5.0 "/6, Gateway Out3/1
  Valid/1 -> "Complex Multiplier 5.0 "/1, Gateway Out2/1
  Convert1/1 -> I/1
  Convert2/1 -> Q/1
  "Complex Multiplier 5.0 "/1 -> valid/1, Gateway Out5/1
  DDS Compiler 4.0/3 -> "Complex Multiplier 5.0 "/7
  Logical2/1 -> DDS Compiler 4.0/3, Gateway Out17/1
  [RX_GLOBAL_RESET] -> Logical2/2
  Convert3/1 -> DDS Compiler 4.0/2
  Constant5/1 -> "Complex Multiplier 5.0 "/5
  tlast/1 -> Convert4/1
  "Complex Multiplier 5.0 "/4 -> Gateway Out7/1, Convert5/1
  Gateway Out5/1 -> CFO Corr/6
  Gateway Out6/1 -> CFO Corr/7
  Gateway Out7/1 -> CFO Corr/8
  Convert4/1 -> "Complex Multiplier 5.0 "/4, Gateway Out4/1
  Convert5/1 -> " tlast"/1
  Sim Reset/1(Out1) -> Logical2/3

== system wlan_phy_rx_pmd/CFO & Samp Buffer/CFO Estimation ==
  note: (1/2) for normalization of phase
      | (1/64) for number of summed elements
  LTS_Sync : Inport
  rx_I : Inport  Port=2
  rx_Q : Inport  Port=3
  rx_valid : Inport  Port=4
  CFO Est : Scope  NumInputPorts=10
      settings: TimeRange=10000  YMin=-1~-1~-0.25~-1~-10~-2.5~-1~0~0~-1  YMax=1~1~2~2~50~15~1~1~0.001~1
  Conj Mult : Subsystem
  Constant : xbsIndex_r4/Constant  const=-1e-4  gui_display_data_type=Fixed-point  arith_type=Signed (2's comp)  n_bits=21  bin_pt=20  preci_type=Single  exp_width=8  frac_width=24  explicit_period=on  period=1  equ=P=C  opselect=C  inp2=PCIN>>17  opr=+  inp1=P  carry=CIN  dbl_ovrd=off  block_type=constant
  Convert2 : xbsIndex_r4/Convert  gui_display_data_type=Fixed-point  arith_type="Signed  (2's comp)"  n_bits=21  bin_pt=13  float_type=Single  exp_bits=8  fraction_bits=24  quantization=Truncate  overflow=Flag as error  en=off  latency=0  dbl_ovrd=off  pipeline=off  block_type=convert
  Delay : xbsIndex_r4/Delay  rst=off  infoeditControl=Selection of Reset will increase slice count due to use of real FFs and instead of SRLs  en=off  latency=8  dbl_ovrd=off  reg_retiming=off  block_type=delay
  Delay1 : xbsIndex_r4/Delay  rst=off  infoeditControl=Selection of Reset will increase slice count due to use of real FFs and instead of SRLs  en=on  latency=64  dbl_ovrd=off  reg_retiming=off  block_type=delay
      settings: xl_area=[7 14 0 14 0 0 0]  block_version=VER_STRING_GOES_HERE
  Delay2 : xbsIndex_r4/Delay  rst=off  infoeditControl=Selection of Reset will increase slice count due to use of real FFs and instead of SRLs  en=on  latency=64  dbl_ovrd=off  reg_retiming=off  block_type=delay
      settings: xl_area=[7 14 0 14 0 0 0]  block_version=VER_STRING_GOES_HERE
  Delay3 : xbsIndex_r4/Delay  rst=off  infoeditControl=Selection of Reset will increase slice count due to use of real FFs and instead of SRLs  en=on  latency=60  dbl_ovrd=off  reg_retiming=off  block_type=delay
      settings: xl_area=[7 14 0 14 0 0 0]  block_version=VER_STRING_GOES_HERE
  Gateway Out{,1..8} (9 alike) : xbsIndex_r4/Gateway Out  inherit_from_input=off  hdl_port=off  timing_constraint=None  locs_specified=off  LOCs={}  UseAsDAC=off  DACChannel='1'  block_type=gatewayout
  Phase : Subsystem
  Posedge3 : Subsystem
  Register : xbsIndex_r4/Register  init=0  rst=on  en=on  dbl_ovrd=off  block_type=register
  Running Sum : Subsystem
  Running Sum1 : Subsystem
  S-R Latch : Subsystem
  Scale : xbsIndex_r4/Scale  scale_factor=-7  dbl_ovrd=off  block_type=scale
      settings: block_version=10.1.2
  CFO : Outport
  tag scope: regRx_CFO_Estimate=global
  wires:
  Gateway Out5/1 -> CFO Est/6  "Sum Q"
  Gateway Out4/1 -> CFO Est/5  "Sum I"
  Gateway Out3/1 -> CFO Est/4  "Conj Prod Q"
  Gateway Out2/1 -> CFO Est/3  "Conj Prod I"
  Gateway Out1/1 -> CFO Est/2  "ADC Q"
  Gateway Out/1 -> CFO Est/1  "ADC I"
  rx_valid/1 -> Conj Mult/1(Valid), Delay2/2, Delay1/2, Delay3/2
  rx_I/1 -> Conj Mult/2(A I), Delay2/1, Gateway Out/1
  rx_Q/1 -> Conj Mult/3(A Q), Delay1/1, Gateway Out1/1
  Delay2/1 -> Conj Mult/4(B I)
  Delay1/1 -> Conj Mult/5(B Q)
  Conj Mult/2(" I") -> Running Sum/2(D), Gateway Out2/1
  Conj Mult/1(" Valid") -> Running Sum/1(En), Running Sum1/1(En)
  Conj Mult/3(" Q") -> Running Sum1/2(D), Gateway Out3/1
  Running Sum/1(Valid) -> Phase/1(Valid)
  Phase/2(Phase) -> Gateway Out6/1, Register/1
  Gateway Out6/1 -> CFO Est/7  "Phase(Sum)"
  Register/1 -> Convert2/1
  Convert2/1 -> Scale/1
  Scale/1 -> Gateway Out8/1, [regRx_CFO_Estimate], CFO/1
  Gateway Out7/1 -> CFO Est/8  "LTS Sync"
  LTS_Sync/1 -> S-R Latch/1(S)
  Gateway Out8/1 -> CFO Est/9  "CFO Est Out"
  ? -> CFO Est/10
  Delay3/1 -> Posedge3/1(D)
  S-R Latch/1(Q) -> Delay/1, Delay3/1
  Delay/1 -> S-R Latch/2(R)
  Posedge3/1(Q) -> Gateway Out7/1, Register/3
  Running Sum/2(Sum) -> Phase/2(Re), Gateway Out4/1
  Running Sum1/2(Sum) -> Phase/3(Im), Gateway Out5/1
  [regRx_BYPASS_CFO_EST] -> Register/2
  Constant/1 -> ?

== system wlan_phy_rx_pmd/CFO & Samp Buffer/FIFO ==
  note: AND not strictly necessary, since FFT
      | only honors tlast when tvalid is asserted.
      | Explicit AND here makes scopes
      | much easier to understand.
  note: This FIFO aids in synchronizing sample generation
      | and feeding the FFT. It would be possible to design
      | the sample buffer control logic not to require
      | this FIFO, but then the logic would need tweaking
      | if the FFT timing or sample processing timing
      | ever change. This FIFO isolates those blocks,
      | at the cost of a little memory and 1 clock cyle latency
      | (assuming the FFT consumes samples faster than
      | they're received)
  data tvalid : Inport
  I : Inport  Port=2
  Q : Inport  Port=3
  data tlast : Inport  Port=4
  FFT tready : Inport  Port=5
  AXI FIFO : xbsIndex_r4/AXI FIFO  input_depth_axis=128  actual_fifo_depth_label=130  enable_tdata=on  enable_tdest=off  enable_tstrb=off  enable_tready=on  enable_tid=off  enable_tuser=off  enable_tkeep=off  enable_tlast=on  has_aresetn=off  enable_data_counts_axis=on  fifo_implementation_type_axis=Common Clock Block RAM  trim_axipin_name=on  programmable_full_type_axis=Full  full_threshold_assert_value_axis=0  programmable_empty_type_axis=Empty  empty_threshold_assert_value_axis=1022  block_type=axi_fifo
  FIFO : Scope  NumInputPorts=9
      settings: TimeRange=14580  YMin=0.95~-1~-1~-1~0.95~-1~-1~-1~-1  YMax=1.05~1~1~1~1.05~1~1~1~1  SaveName=ScopeData20
  Gateway Out{1..6,8..9} (8 alike) : xbsIndex_r4/Gateway Out  inherit_from_input=off  hdl_port=off  timing_constraint=None  locs_specified=off  LOCs={}  UseAsDAC=off  DACChannel='1'  block_type=gatewayout
  I/Q Concat : Subsystem
  I/Q Slice : Subsystem
  Logical2 : xbsIndex_r4/Logical  logical_function=AND  inputs=2  en=off  latency=0  precision=Full  arith_type=Unsigned  n_bits=16  bin_pt=0  align_bp=on  dbl_ovrd=off  block_type=logical
  FFT tvalid : Outport
  FFT tdata_re : Outport  Port=2
  FFT tdata_im : Outport  Port=3
  FFT tlast : Outport  Port=4
  FIFO tready : Outport  Port=5
  wires:
  AXI FIFO/2 -> I/Q Slice/1(IQ)
  AXI FIFO/1 -> FFT tvalid/1, Gateway Out1/1, Logical2/1
  AXI FIFO/3 -> Logical2/2
  Gateway Out8/1 -> FIFO/1  "FFT ready"
  I/Q Slice/1(I) -> FFT tdata_re/1
  I/Q Slice/2(Q) -> FFT tdata_im/1, Gateway Out2/1
  FFT tready/1 -> Gateway Out8/1, AXI FIFO/1
  data tvalid/1 -> Gateway Out5/1, AXI FIFO/2
  data tlast/1 -> Gateway Out6/1, AXI FIFO/4
  AXI FIFO/4 -> FIFO tready/1, Gateway Out4/1
  Gateway Out1/1 -> FIFO/2  "FIFO out valid"
  Gateway Out2/1 -> FIFO/3  "Q out"
  Gateway Out3/1 -> FIFO/4  "tlast out"
  Gateway Out4/1 -> FIFO/5  "FIFO ready"
  Gateway Out5/1 -> FIFO/6  "valid in"
  Gateway Out6/1 -> FIFO/7  "tlast in"
  Logical2/1 -> FFT tlast/1, Gateway Out3/1
  I/1 -> I/Q Concat/1(I)
  Q/1 -> I/Q Concat/2(Q)
  I/Q Concat/1(IQ) -> AXI FIFO/3
  ? -> FIFO/8  "Reset"
  Gateway Out9/1 -> FIFO/9  "FIFO Occ"
  AXI FIFO/5 -> Gateway Out9/1
